simulink model "HvacMdlAirMFlowFrnt"  (R2022b)
settings: Solver=FixedStepDiscrete  StartTime=0.0  StopTime=10.0  FixedStep=0.2  RelTol=1e-3
settings Model: Creator=blue.ge  LastModifiedBy=blue.ge
settings DataIOCC: SaveFormat=StructureWithTime
settings OptimizationCC: DefaultParameterBehavior=Inlined  EnhancedBackFolding=on  PassReuseOutputArgsAs=Individual arguments  ZeroExternalMemoryAtStartup=off  ZeroInternalMemoryAtStartup=off  OptimizeBlockOrder=speed  DifferentSizesBufferReuse=on  OptimizationCustomize=off  EfficientTunableParamExpr=on
settings DebuggingCC: ParameterTunabilityLossMsg=error
settings ModelReferenceCC: ModelReferenceNumInstancesAllowed=Single
settings SFSimCC: MATLABDynamicMemAlloc=off
settings RTWCC: Description=AUTOSAR  SystemTargetFile=autosar.tlc  GenCodeOnly=on  TemplateMakefile=ert_default_tmf
settings CodeAppCC: ForceParamTrailComments=on  IgnoreCustomStorageClasses=off  OperatorAnnotations=on  SimulinkDataObjDesc=on  SFDataObjDesc=on  InsertBlockDesc=on
settings STFCustomTargetCC: TargetLangStandard=C99 (ISO)  MultiwordLength=256  ParMdlRefBuildCompliant=on  CompOptLevelCompliant=on  IncludeMdlTerminateFcn=off  CombineOutputUpdateFcns=on  SuppressErrorStatus=on  ERTCustomFileBanners=on  SupportNonFinite=off  SupportComplex=off  SupportContinuousTime=off  SupportNonInlinedSFcns=off  RemoveResetFunc=on  MATLABClassNameForMDSCustomization=Simulink.SoftwareTarget.ERTCustomization  AutosarCompliant=on  UseToolchainInfoCompliant=on  LookupTableObjectStructAxisOrder=2,1,3,4,...  LUTObjectStructOrderEvenSpacing=Size,Table,Breakpoints  SystemTargetFile=autosar.tlc
settings ERTTargetCC: TargetLangStandard=C99 (ISO)  ConcurrentExecutionCompliant=off  IncludeMdlTerminateFcn=off  SuppressErrorStatus=on  SupportNonFinite=off  SupportComplex=off  ModelStepFunctionPrototypeControlCompliant=off  CPPClassGenCompliant=off  AutosarCompliant=on  LookupTableObjectStructAxisOrder=2,1,3,4,...  LUTObjectStructOrderEvenSpacing=Size,Table,Breakpoints  InlinedParameterPlacement=NonHierarchical  ERTCustomFileTemplate=example_file_process.tlc

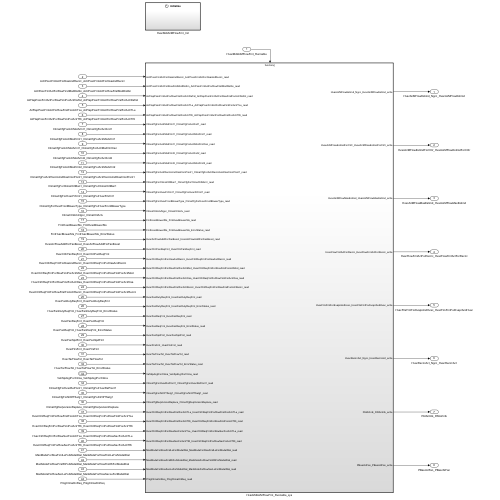
[thumbnail: Simulink's stored preview of the top-level diagram]
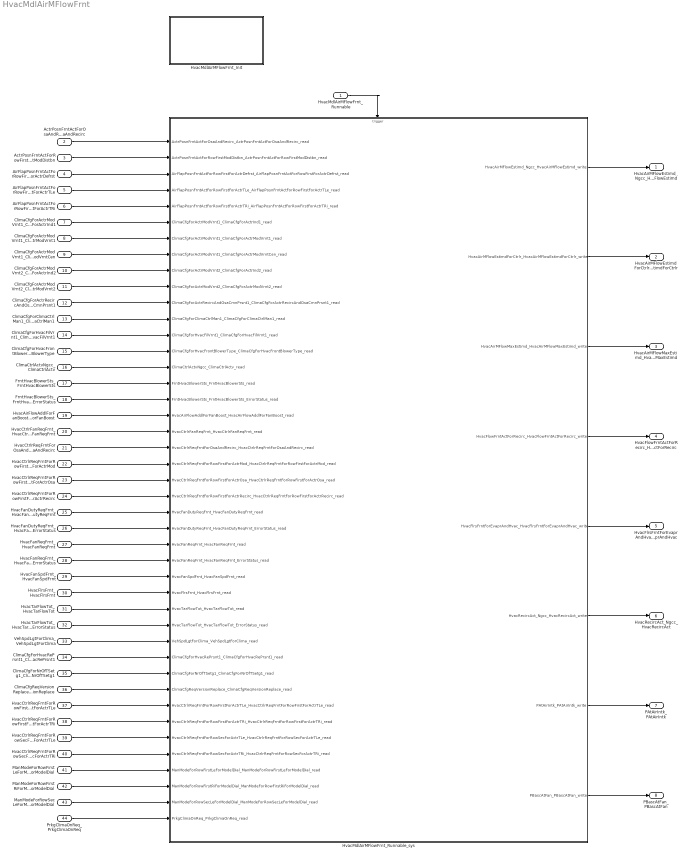
== system HvacMdlAirMFlowFrnt ==
  HvacMdlAirMFlowFrnt_Runnable : Inport  SampleTime=0.2  OutputFunctionCall=on
  ActrPosnFrntActForOsaAndRecirc_ActrPosnFrntActForOsaAndRecirc : Inport  Port=2  OutMin=0  OutMax=100  OutDataTypeStr=Perc9  PortDimensions=1
  ActrPosnFrntActForRowFirstModDistbn_ActrPosnFrntActForRowFirstModDistbn : Inport  Port=3  OutMin=0  OutMax=100  OutDataTypeStr=Perc9  PortDimensions=1
  AirFlapPosnFrntActForRowFirstForActrDefrst_AirFlapPosnFrntActForRowFirstForActrDefrst : Inport  Port=4  OutMin=0  OutMax=100  OutDataTypeStr=Perc9  PortDimensions=1
  AirFlapPosnFrntActForRowFirstForActrTLe_AirFlapPosnFrntActForRowFirstForActrTLe : Inport  Port=5  OutMin=0  OutMax=100  OutDataTypeStr=Perc9  PortDimensions=1
  AirFlapPosnFrntActForRowFirstForActrTRi_AirFlapPosnFrntActForRowFirstForActrTRi : Inport  Port=6  OutMin=0  OutMax=100  OutDataTypeStr=Perc9  PortDimensions=1
  ClimaCfgForActrModVrnt1_ClimaCfgForActrInd1 : Inport  Port=7  OutDataTypeStr=Enum: ClimaCfgForActrInd1  PortDimensions=1
  ClimaCfgForActrModVrnt1_ClimaCfgForActrModVrnt1 : Inport  Port=8  OutDataTypeStr=Enum: ClimaCfgForActrModVrnt1  PortDimensions=1
  ClimaCfgForActrModVrnt1_ClimaCfgForActrModVrntCen : Inport  Port=9  OutDataTypeStr=Enum: ClimaCfgForActrModVrntCen  PortDimensions=1
  ClimaCfgForActrModVrnt2_ClimaCfgForActrInd2 : Inport  Port=10  OutDataTypeStr=Enum: ClimaCfgForActrInd2  PortDimensions=1
  ClimaCfgForActrModVrnt2_ClimaCfgForActrModVrnt2 : Inport  Port=11  OutDataTypeStr=Enum: ClimaCfgForActrModVrnt2  PortDimensions=1
  ClimaCfgForActrRecircAndOsaCmnPrsnt1_ClimaCfgForActrRecircAndOsaCmnPrsnt1 : Inport  Port=12  OutDataTypeStr=Enum: ClimaCfgForActrRecircAndOsaCmnPrsnt1  PortDimensions=1
  ClimaCfgForClimaCtrlMan1_ClimaCfgForClimaCtrlMan1 : Inport  Port=13  OutDataTypeStr=Boolean  PortDimensions=1
  ClimaCfgForHvacFilVrnt1_ClimaCfgForHvacFilVrnt1 : Inport  Port=14  OutDataTypeStr=Enum: CarCfgForHvacFilTypGly  PortDimensions=1
  ClimaCfgForHvacFrontBlowerType_ClimaCfgForHvacFrontBlowerType : Inport  Port=15  OutDataTypeStr=Enum: ClimaCfgForHvacFrontBlowerType  PortDimensions=1
  ClimaCtrlActvNgcc_ClimaCtrlActv : Inport  Port=16  OutDataTypeStr=Boolean  PortDimensions=1
  FrntHvacBlowerSts_FrntHvacBlowerSts : Inport  Port=17  OutDataTypeStr=Enum: HvacFanSts  PortDimensions=1
  FrntHvacBlowerSts_FrntHvacBlowerSts_ErrorStatus : Inport  Port=18  OutDataTypeStr=uint8  PortDimensions=1
  HvacAirFlowAddlForFanBoost_HvacAirFlowAddlForFanBoost : Inport  Port=19  OutDataTypeStr=AirFlow30  PortDimensions=1
  HvacCtrlrFanReqFrnt_HvacCtrlrFanReqFrnt : Inport  Port=20  OutDataTypeStr=FanSpd1  PortDimensions=1
  HvacCtrlrReqFrntForOsaAndRecirc_HvacCtrlrReqFrntForOsaAndRecirc : Inport  Port=21  OutDataTypeStr=Perc30  PortDimensions=1
  HvacCtrlrReqFrntForRowFirstForActrMod_HvacCtrlrReqFrntForRowFirstForActrMod : Inport  Port=22  OutDataTypeStr=Perc30  PortDimensions=1
  HvacCtrlrReqFrntForRowFirstForActrOsa_HvacCtrlrReqFrntForRowFirstForActrOsa : Inport  Port=23  OutDataTypeStr=Perc30  PortDimensions=1
  HvacCtrlrReqFrntForRowFirstForActrRecirc_HvacCtrlrReqFrntForRowFirstForActrRecirc : Inport  Port=24  OutDataTypeStr=Perc30  PortDimensions=1
  HvacFanDutyReqFrnt_HvacFanDutyReqFrnt : Inport  Port=25  OutMin=0  OutMax=100  OutDataTypeStr=Perc9  PortDimensions=1
  HvacFanDutyReqFrnt_HvacFanDutyReqFrnt_ErrorStatus : Inport  Port=26  OutDataTypeStr=uint8  PortDimensions=1
  HvacFanReqFrnt_HvacFanReqFrnt : Inport  Port=27  OutDataTypeStr=HvacFanSpd  PortDimensions=1
  HvacFanReqFrnt_HvacFanReqFrnt_ErrorStatus : Inport  Port=28  OutDataTypeStr=uint8  PortDimensions=1
  HvacFanSpdFrnt_HvacFanSpdFrnt : Inport  Port=29  OutDataTypeStr=HvacFanSpd  PortDimensions=1
  HvacFlrsFrnt_HvacFlrsFrnt : Inport  Port=30  OutDataTypeStr=Flrs30  PortDimensions=1
  HvacTarFlowTot_HvacTarFlowTot : Inport  Port=31  OutDataTypeStr=AirFlow30  PortDimensions=1
  HvacTarFlowTot_HvacTarFlowTot_ErrorStatus : Inport  Port=32  OutDataTypeStr=uint8  PortDimensions=1
  VehSpdLgtForClima_VehSpdLgtForClima : Inport  Port=33  OutDataTypeStr=Bus: VehSpdLgtForClima  PortDimensions=1  BusOutputAsStruct=on
  ClimaCfgForHvacRePrsnt1_ClimaCfgForHvacRePrsnt1 : Inport  Port=34  OutDataTypeStr=Enum: CarCfgForHvacRe  PortDimensions=1
  ClimaCfgForNrOfTSetg1_ClimaCfgForNrOfTSetg1 : Inport  Port=35  OutDataTypeStr=Enum: ClimaCfgForNrOfTSetg1  PortDimensions=1
  ClimaCfgReqVersionReplace_ClimaCfgReqVersionReplace : Inport  Port=36  OutDataTypeStr=UInt8  PortDimensions=1
  HvacCtrlrReqFrntForRowFirstForActrTLe_HvacCtrlrReqFrntForRowFirstForActrTLe : Inport  Port=37  OutDataTypeStr=Perc30  PortDimensions=1
  HvacCtrlrReqFrntForRowFirstForActrTRi_HvacCtrlrReqFrntForRowFirstForActrTRi : Inport  Port=38  OutDataTypeStr=Perc30  PortDimensions=1
  HvacCtrlrReqFrntForRowSecForActrTLe_HvacCtrlrReqFrntForRowSecForActrTLe : Inport  Port=39  OutDataTypeStr=Perc30  PortDimensions=1
  HvacCtrlrReqFrntForRowSecForActrTRi_HvacCtrlrReqFrntForRowSecForActrTRi : Inport  Port=40  OutDataTypeStr=Perc30  PortDimensions=1
  ManModeForRowFirstLeForModelDial_ManModeForRowFirstLeForModelDial : Inport  Port=41  OutDataTypeStr=Enum: HmiCmptmtAirDistbnFrnt  PortDimensions=1
  ManModeForRowFirstRiForModelDial_ManModeForRowFirstRiForModelDial : Inport  Port=42  OutDataTypeStr=Enum: HmiCmptmtAirDistbnFrnt  PortDimensions=1
  ManModeForRowSecLeForModelDial_ManModeForRowSecLeForModelDial : Inport  Port=43  OutDataTypeStr=Enum: HmiCmptmtAirDistbnFrnt  PortDimensions=1
  PrkgClimaOnReq_PrkgClimaOnReq : Inport  Port=44  OutDataTypeStr=Boolean  PortDimensions=1
  HvacMdlAirMFlowFrnt_Init : Subsystem
  HvacMdlAirMFlowFrnt_Runnable_sys : Subsystem
  HvacAirMFlowEstimd_Ngcc_HvacAirMFlowEstimd : Outport  OutMin=0  OutMax=1000  OutDataTypeStr=MFlow1  PortDimensions=1
  HvacAirMFlowEstimdForCtrlr_HvacAirMFlowEstimdForCtrlr : Outport  Port=2  OutDataTypeStr=AirFlow30  PortDimensions=1
  HvacAirMFlowMaxEstimd_HvacAirMFlowMaxEstimd : Outport  Port=3  OutDataTypeStr=AirFlow30  PortDimensions=1
  HvacFlowFrntActForRecirc_HvacFlowFrntActForRecirc : Outport  Port=4  OutDataTypeStr=AirFlow30  PortDimensions=1
  HvacFlrsFrntForEvaprAndHvac_HvacFlrsFrntForEvaprAndHvac : Outport  Port=5  OutDataTypeStr=Flrs30  PortDimensions=1
  HvacRecircAct_Ngcc_HvacRecircAct : Outport  Port=6  OutDataTypeStr=Perc4  PortDimensions=1
  PAtAirIntk_PAtAirIntk : Outport  Port=7  OutDataTypeStr=AirP31  PortDimensions=1
  PBascAtFan_PBascAtFan : Outport  Port=8  OutDataTypeStr=AirP31  PortDimensions=1
  wires:
  ActrPosnFrntActForOsaAndRecirc_ActrPosnFrntActForOsaAndRecirc/1 -> HvacMdlAirMFlowFrnt_Runnable_sys/1(ActrPosnFrntActForOsaAndRecirc_ActrPosnFrntActForOsaAndRecirc_read)
  ActrPosnFrntActForRowFirstModDistbn_ActrPosnFrntActForRowFirstModDistbn/1 -> HvacMdlAirMFlowFrnt_Runnable_sys/2(ActrPosnFrntActForRowFirstModDistbn_ActrPosnFrntActForRowFirstModDistbn_read)
  AirFlapPosnFrntActForRowFirstForActrDefrst_AirFlapPosnFrntActForRowFirstForActrDefrst/1 -> HvacMdlAirMFlowFrnt_Runnable_sys/3(AirFlapPosnFrntActForRowFirstForActrDefrst_AirFlapPosnFrntActForRowFirstForActrDefrst_read)
  AirFlapPosnFrntActForRowFirstForActrTLe_AirFlapPosnFrntActForRowFirstForActrTLe/1 -> HvacMdlAirMFlowFrnt_Runnable_sys/4(AirFlapPosnFrntActForRowFirstForActrTLe_AirFlapPosnFrntActForRowFirstForActrTLe_read)
  AirFlapPosnFrntActForRowFirstForActrTRi_AirFlapPosnFrntActForRowFirstForActrTRi/1 -> HvacMdlAirMFlowFrnt_Runnable_sys/5(AirFlapPosnFrntActForRowFirstForActrTRi_AirFlapPosnFrntActForRowFirstForActrTRi_read)
  ClimaCfgForActrModVrnt1_ClimaCfgForActrInd1/1 -> HvacMdlAirMFlowFrnt_Runnable_sys/6(ClimaCfgForActrModVrnt1_ClimaCfgForActrInd1_read)
  ClimaCfgForActrModVrnt1_ClimaCfgForActrModVrnt1/1 -> HvacMdlAirMFlowFrnt_Runnable_sys/7(ClimaCfgForActrModVrnt1_ClimaCfgForActrModVrnt1_read)
  ClimaCfgForActrModVrnt1_ClimaCfgForActrModVrntCen/1 -> HvacMdlAirMFlowFrnt_Runnable_sys/8(ClimaCfgForActrModVrnt1_ClimaCfgForActrModVrntCen_read)
  ClimaCfgForActrModVrnt2_ClimaCfgForActrInd2/1 -> HvacMdlAirMFlowFrnt_Runnable_sys/9(ClimaCfgForActrModVrnt2_ClimaCfgForActrInd2_read)
  ClimaCfgForActrModVrnt2_ClimaCfgForActrModVrnt2/1 -> HvacMdlAirMFlowFrnt_Runnable_sys/10(ClimaCfgForActrModVrnt2_ClimaCfgForActrModVrnt2_read)
  ClimaCfgForActrRecircAndOsaCmnPrsnt1_ClimaCfgForActrRecircAndOsaCmnPrsnt1/1 -> HvacMdlAirMFlowFrnt_Runnable_sys/11(ClimaCfgForActrRecircAndOsaCmnPrsnt1_ClimaCfgForActrRecircAndOsaCmnPrsnt1_read)
  ClimaCfgForClimaCtrlMan1_ClimaCfgForClimaCtrlMan1/1 -> HvacMdlAirMFlowFrnt_Runnable_sys/12(ClimaCfgForClimaCtrlMan1_ClimaCfgForClimaCtrlMan1_read)
  ClimaCfgForHvacFilVrnt1_ClimaCfgForHvacFilVrnt1/1 -> HvacMdlAirMFlowFrnt_Runnable_sys/13(ClimaCfgForHvacFilVrnt1_ClimaCfgForHvacFilVrnt1_read)
  ClimaCfgForHvacFrontBlowerType_ClimaCfgForHvacFrontBlowerType/1 -> HvacMdlAirMFlowFrnt_Runnable_sys/14(ClimaCfgForHvacFrontBlowerType_ClimaCfgForHvacFrontBlowerType_read)
  ClimaCtrlActvNgcc_ClimaCtrlActv/1 -> HvacMdlAirMFlowFrnt_Runnable_sys/15(ClimaCtrlActvNgcc_ClimaCtrlActv_read)
  FrntHvacBlowerSts_FrntHvacBlowerSts/1 -> HvacMdlAirMFlowFrnt_Runnable_sys/16(FrntHvacBlowerSts_FrntHvacBlowerSts_read)
  FrntHvacBlowerSts_FrntHvacBlowerSts_ErrorStatus/1 -> HvacMdlAirMFlowFrnt_Runnable_sys/17(FrntHvacBlowerSts_FrntHvacBlowerSts_ErrorStatus_read)
  HvacAirFlowAddlForFanBoost_HvacAirFlowAddlForFanBoost/1 -> HvacMdlAirMFlowFrnt_Runnable_sys/18(HvacAirFlowAddlForFanBoost_HvacAirFlowAddlForFanBoost_read)
  HvacCtrlrFanReqFrnt_HvacCtrlrFanReqFrnt/1 -> HvacMdlAirMFlowFrnt_Runnable_sys/19(HvacCtrlrFanReqFrnt_HvacCtrlrFanReqFrnt_read)
  HvacCtrlrReqFrntForOsaAndRecirc_HvacCtrlrReqFrntForOsaAndRecirc/1 -> HvacMdlAirMFlowFrnt_Runnable_sys/20(HvacCtrlrReqFrntForOsaAndRecirc_HvacCtrlrReqFrntForOsaAndRecirc_read)
  HvacCtrlrReqFrntForRowFirstForActrMod_HvacCtrlrReqFrntForRowFirstForActrMod/1 -> HvacMdlAirMFlowFrnt_Runnable_sys/21(HvacCtrlrReqFrntForRowFirstForActrMod_HvacCtrlrReqFrntForRowFirstForActrMod_read)
  HvacCtrlrReqFrntForRowFirstForActrOsa_HvacCtrlrReqFrntForRowFirstForActrOsa/1 -> HvacMdlAirMFlowFrnt_Runnable_sys/22(HvacCtrlrReqFrntForRowFirstForActrOsa_HvacCtrlrReqFrntForRowFirstForActrOsa_read)
  HvacCtrlrReqFrntForRowFirstForActrRecirc_HvacCtrlrReqFrntForRowFirstForActrRecirc/1 -> HvacMdlAirMFlowFrnt_Runnable_sys/23(HvacCtrlrReqFrntForRowFirstForActrRecirc_HvacCtrlrReqFrntForRowFirstForActrRecirc_read)
  HvacFanDutyReqFrnt_HvacFanDutyReqFrnt/1 -> HvacMdlAirMFlowFrnt_Runnable_sys/24(HvacFanDutyReqFrnt_HvacFanDutyReqFrnt_read)
  HvacFanDutyReqFrnt_HvacFanDutyReqFrnt_ErrorStatus/1 -> HvacMdlAirMFlowFrnt_Runnable_sys/25(HvacFanDutyReqFrnt_HvacFanDutyReqFrnt_ErrorStatus_read)
  HvacFanReqFrnt_HvacFanReqFrnt/1 -> HvacMdlAirMFlowFrnt_Runnable_sys/26(HvacFanReqFrnt_HvacFanReqFrnt_read)
  HvacFanReqFrnt_HvacFanReqFrnt_ErrorStatus/1 -> HvacMdlAirMFlowFrnt_Runnable_sys/27(HvacFanReqFrnt_HvacFanReqFrnt_ErrorStatus_read)
  HvacFanSpdFrnt_HvacFanSpdFrnt/1 -> HvacMdlAirMFlowFrnt_Runnable_sys/28(HvacFanSpdFrnt_HvacFanSpdFrnt_read)
  HvacFlrsFrnt_HvacFlrsFrnt/1 -> HvacMdlAirMFlowFrnt_Runnable_sys/29(HvacFlrsFrnt_HvacFlrsFrnt_read)
  HvacTarFlowTot_HvacTarFlowTot/1 -> HvacMdlAirMFlowFrnt_Runnable_sys/30(HvacTarFlowTot_HvacTarFlowTot_read)
  HvacTarFlowTot_HvacTarFlowTot_ErrorStatus/1 -> HvacMdlAirMFlowFrnt_Runnable_sys/31(HvacTarFlowTot_HvacTarFlowTot_ErrorStatus_read)
  VehSpdLgtForClima_VehSpdLgtForClima/1 -> HvacMdlAirMFlowFrnt_Runnable_sys/32(VehSpdLgtForClima_VehSpdLgtForClima_read)
  ClimaCfgForHvacRePrsnt1_ClimaCfgForHvacRePrsnt1/1 -> HvacMdlAirMFlowFrnt_Runnable_sys/33(ClimaCfgForHvacRePrsnt1_ClimaCfgForHvacRePrsnt1_read)
  ClimaCfgForNrOfTSetg1_ClimaCfgForNrOfTSetg1/1 -> HvacMdlAirMFlowFrnt_Runnable_sys/34(ClimaCfgForNrOfTSetg1_ClimaCfgForNrOfTSetg1_read)
  ClimaCfgReqVersionReplace_ClimaCfgReqVersionReplace/1 -> HvacMdlAirMFlowFrnt_Runnable_sys/35(ClimaCfgReqVersionReplace_ClimaCfgReqVersionReplace_read)
  HvacCtrlrReqFrntForRowFirstForActrTLe_HvacCtrlrReqFrntForRowFirstForActrTLe/1 -> HvacMdlAirMFlowFrnt_Runnable_sys/36(HvacCtrlrReqFrntForRowFirstForActrTLe_HvacCtrlrReqFrntForRowFirstForActrTLe_read)
  HvacCtrlrReqFrntForRowFirstForActrTRi_HvacCtrlrReqFrntForRowFirstForActrTRi/1 -> HvacMdlAirMFlowFrnt_Runnable_sys/37(HvacCtrlrReqFrntForRowFirstForActrTRi_HvacCtrlrReqFrntForRowFirstForActrTRi_read)
  HvacCtrlrReqFrntForRowSecForActrTLe_HvacCtrlrReqFrntForRowSecForActrTLe/1 -> HvacMdlAirMFlowFrnt_Runnable_sys/38(HvacCtrlrReqFrntForRowSecForActrTLe_HvacCtrlrReqFrntForRowSecForActrTLe_read)
  HvacCtrlrReqFrntForRowSecForActrTRi_HvacCtrlrReqFrntForRowSecForActrTRi/1 -> HvacMdlAirMFlowFrnt_Runnable_sys/39(HvacCtrlrReqFrntForRowSecForActrTRi_HvacCtrlrReqFrntForRowSecForActrTRi_read)
  ManModeForRowFirstLeForModelDial_ManModeForRowFirstLeForModelDial/1 -> HvacMdlAirMFlowFrnt_Runnable_sys/40(ManModeForRowFirstLeForModelDial_ManModeForRowFirstLeForModelDial_read)
  ManModeForRowFirstRiForModelDial_ManModeForRowFirstRiForModelDial/1 -> HvacMdlAirMFlowFrnt_Runnable_sys/41(ManModeForRowFirstRiForModelDial_ManModeForRowFirstRiForModelDial_read)
  ManModeForRowSecLeForModelDial_ManModeForRowSecLeForModelDial/1 -> HvacMdlAirMFlowFrnt_Runnable_sys/42(ManModeForRowSecLeForModelDial_ManModeForRowSecLeForModelDial_read)
  PrkgClimaOnReq_PrkgClimaOnReq/1 -> HvacMdlAirMFlowFrnt_Runnable_sys/43(PrkgClimaOnReq_PrkgClimaOnReq_read)
  HvacMdlAirMFlowFrnt_Runnable_sys/1(HvacAirMFlowEstimd_Ngcc_HvacAirMFlowEstimd_write) -> HvacAirMFlowEstimd_Ngcc_HvacAirMFlowEstimd/1
  HvacMdlAirMFlowFrnt_Runnable_sys/2(HvacAirMFlowEstimdForCtrlr_HvacAirMFlowEstimdForCtrlr_write) -> HvacAirMFlowEstimdForCtrlr_HvacAirMFlowEstimdForCtrlr/1
  HvacMdlAirMFlowFrnt_Runnable_sys/3(HvacAirMFlowMaxEstimd_HvacAirMFlowMaxEstimd_write) -> HvacAirMFlowMaxEstimd_HvacAirMFlowMaxEstimd/1
  HvacMdlAirMFlowFrnt_Runnable_sys/4(HvacFlowFrntActForRecirc_HvacFlowFrntActForRecirc_write) -> HvacFlowFrntActForRecirc_HvacFlowFrntActForRecirc/1
  HvacMdlAirMFlowFrnt_Runnable_sys/5(HvacFlrsFrntForEvaprAndHvac_HvacFlrsFrntForEvaprAndHvac_write) -> HvacFlrsFrntForEvaprAndHvac_HvacFlrsFrntForEvaprAndHvac/1
  HvacMdlAirMFlowFrnt_Runnable_sys/6(HvacRecircAct_Ngcc_HvacRecircAct_write) -> HvacRecircAct_Ngcc_HvacRecircAct/1
  HvacMdlAirMFlowFrnt_Runnable_sys/7(PAtAirIntk_PAtAirIntk_write) -> PAtAirIntk_PAtAirIntk/1
  HvacMdlAirMFlowFrnt_Runnable_sys/8(PBascAtFan_PBascAtFan_write) -> PBascAtFan_PBascAtFan/1
  HvacMdlAirMFlowFrnt_Runnable/1 -> HvacMdlAirMFlowFrnt_Runnable_sys/Trigger

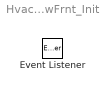
== system HvacMdlAirMFlowFrnt/HvacMdlAirMFlowFrnt_Init ==
  Event Listener : EventListener  EventName=reset

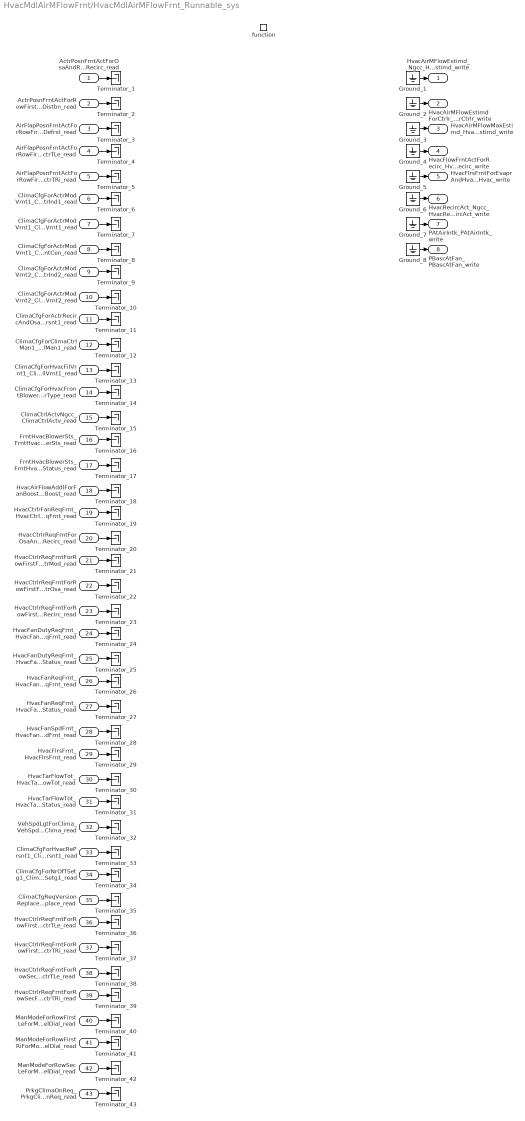
== system HvacMdlAirMFlowFrnt/HvacMdlAirMFlowFrnt_Runnable_sys ==
  ActrPosnFrntActForOsaAndRecirc_ActrPosnFrntActForOsaAndRecirc_read : Inport
  ActrPosnFrntActForRowFirstModDistbn_ActrPosnFrntActForRowFirstModDistbn_read : Inport  Port=2
  AirFlapPosnFrntActForRowFirstForActrDefrst_AirFlapPosnFrntActForRowFirstForActrDefrst_read : Inport  Port=3
  AirFlapPosnFrntActForRowFirstForActrTLe_AirFlapPosnFrntActForRowFirstForActrTLe_read : Inport  Port=4
  AirFlapPosnFrntActForRowFirstForActrTRi_AirFlapPosnFrntActForRowFirstForActrTRi_read : Inport  Port=5
  ClimaCfgForActrModVrnt1_ClimaCfgForActrInd1_read : Inport  Port=6
  ClimaCfgForActrModVrnt1_ClimaCfgForActrModVrnt1_read : Inport  Port=7
  ClimaCfgForActrModVrnt1_ClimaCfgForActrModVrntCen_read : Inport  Port=8
  ClimaCfgForActrModVrnt2_ClimaCfgForActrInd2_read : Inport  Port=9
  ClimaCfgForActrModVrnt2_ClimaCfgForActrModVrnt2_read : Inport  Port=10
  ClimaCfgForActrRecircAndOsaCmnPrsnt1_ClimaCfgForActrRecircAndOsaCmnPrsnt1_read : Inport  Port=11
  ClimaCfgForClimaCtrlMan1_ClimaCfgForClimaCtrlMan1_read : Inport  Port=12
  ClimaCfgForHvacFilVrnt1_ClimaCfgForHvacFilVrnt1_read : Inport  Port=13
  ClimaCfgForHvacFrontBlowerType_ClimaCfgForHvacFrontBlowerType_read : Inport  Port=14
  ClimaCtrlActvNgcc_ClimaCtrlActv_read : Inport  Port=15
  FrntHvacBlowerSts_FrntHvacBlowerSts_read : Inport  Port=16
  FrntHvacBlowerSts_FrntHvacBlowerSts_ErrorStatus_read : Inport  Port=17
  HvacAirFlowAddlForFanBoost_HvacAirFlowAddlForFanBoost_read : Inport  Port=18
  HvacCtrlrFanReqFrnt_HvacCtrlrFanReqFrnt_read : Inport  Port=19
  HvacCtrlrReqFrntForOsaAndRecirc_HvacCtrlrReqFrntForOsaAndRecirc_read : Inport  Port=20
  HvacCtrlrReqFrntForRowFirstForActrMod_HvacCtrlrReqFrntForRowFirstForActrMod_read : Inport  Port=21
  HvacCtrlrReqFrntForRowFirstForActrOsa_HvacCtrlrReqFrntForRowFirstForActrOsa_read : Inport  Port=22
  HvacCtrlrReqFrntForRowFirstForActrRecirc_HvacCtrlrReqFrntForRowFirstForActrRecirc_read : Inport  Port=23
  HvacFanDutyReqFrnt_HvacFanDutyReqFrnt_read : Inport  Port=24
  HvacFanDutyReqFrnt_HvacFanDutyReqFrnt_ErrorStatus_read : Inport  Port=25
  HvacFanReqFrnt_HvacFanReqFrnt_read : Inport  Port=26
  HvacFanReqFrnt_HvacFanReqFrnt_ErrorStatus_read : Inport  Port=27
  HvacFanSpdFrnt_HvacFanSpdFrnt_read : Inport  Port=28
  HvacFlrsFrnt_HvacFlrsFrnt_read : Inport  Port=29
  HvacTarFlowTot_HvacTarFlowTot_read : Inport  Port=30
  HvacTarFlowTot_HvacTarFlowTot_ErrorStatus_read : Inport  Port=31
  VehSpdLgtForClima_VehSpdLgtForClima_read : Inport  Port=32
  ClimaCfgForHvacRePrsnt1_ClimaCfgForHvacRePrsnt1_read : Inport  Port=33
  ClimaCfgForNrOfTSetg1_ClimaCfgForNrOfTSetg1_read : Inport  Port=34
  ClimaCfgReqVersionReplace_ClimaCfgReqVersionReplace_read : Inport  Port=35
  HvacCtrlrReqFrntForRowFirstForActrTLe_HvacCtrlrReqFrntForRowFirstForActrTLe_read : Inport  Port=36
  HvacCtrlrReqFrntForRowFirstForActrTRi_HvacCtrlrReqFrntForRowFirstForActrTRi_read : Inport  Port=37
  HvacCtrlrReqFrntForRowSecForActrTLe_HvacCtrlrReqFrntForRowSecForActrTLe_read : Inport  Port=38
  HvacCtrlrReqFrntForRowSecForActrTRi_HvacCtrlrReqFrntForRowSecForActrTRi_read : Inport  Port=39
  ManModeForRowFirstLeForModelDial_ManModeForRowFirstLeForModelDial_read : Inport  Port=40
  ManModeForRowFirstRiForModelDial_ManModeForRowFirstRiForModelDial_read : Inport  Port=41
  ManModeForRowSecLeForModelDial_ManModeForRowSecLeForModelDial_read : Inport  Port=42
  PrkgClimaOnReq_PrkgClimaOnReq_read : Inport  Port=43
  function : TriggerPort  TriggerType=function-call  VariantControl=(inherit)  SampleTimeType=periodic  SampleTime=-1
  Ground_{1..8} (8 alike) : Ground
  Terminator_{1..43} (43 alike) : Terminator
  HvacAirMFlowEstimd_Ngcc_HvacAirMFlowEstimd_write : Outport
      settings: EnsureOutportIsVirtual=on
  HvacAirMFlowEstimdForCtrlr_HvacAirMFlowEstimdForCtrlr_write : Outport  Port=2
      settings: EnsureOutportIsVirtual=on
  HvacAirMFlowMaxEstimd_HvacAirMFlowMaxEstimd_write : Outport  Port=3
      settings: EnsureOutportIsVirtual=on
  HvacFlowFrntActForRecirc_HvacFlowFrntActForRecirc_write : Outport  Port=4
      settings: EnsureOutportIsVirtual=on
  HvacFlrsFrntForEvaprAndHvac_HvacFlrsFrntForEvaprAndHvac_write : Outport  Port=5
      settings: EnsureOutportIsVirtual=on
  HvacRecircAct_Ngcc_HvacRecircAct_write : Outport  Port=6
      settings: EnsureOutportIsVirtual=on
  PAtAirIntk_PAtAirIntk_write : Outport  Port=7
      settings: EnsureOutportIsVirtual=on
  PBascAtFan_PBascAtFan_write : Outport  Port=8
      settings: EnsureOutportIsVirtual=on
  wires:
  ActrPosnFrntActForOsaAndRecirc_ActrPosnFrntActForOsaAndRecirc_read/1 -> Terminator_1/1
  ActrPosnFrntActForRowFirstModDistbn_ActrPosnFrntActForRowFirstModDistbn_read/1 -> Terminator_2/1
  AirFlapPosnFrntActForRowFirstForActrDefrst_AirFlapPosnFrntActForRowFirstForActrDefrst_read/1 -> Terminator_3/1
  AirFlapPosnFrntActForRowFirstForActrTLe_AirFlapPosnFrntActForRowFirstForActrTLe_read/1 -> Terminator_4/1
  AirFlapPosnFrntActForRowFirstForActrTRi_AirFlapPosnFrntActForRowFirstForActrTRi_read/1 -> Terminator_5/1
  ClimaCfgForActrModVrnt1_ClimaCfgForActrInd1_read/1 -> Terminator_6/1
  ClimaCfgForActrModVrnt1_ClimaCfgForActrModVrnt1_read/1 -> Terminator_7/1
  ClimaCfgForActrModVrnt1_ClimaCfgForActrModVrntCen_read/1 -> Terminator_8/1
  ClimaCfgForActrModVrnt2_ClimaCfgForActrInd2_read/1 -> Terminator_9/1
  ClimaCfgForActrModVrnt2_ClimaCfgForActrModVrnt2_read/1 -> Terminator_10/1
  ClimaCfgForActrRecircAndOsaCmnPrsnt1_ClimaCfgForActrRecircAndOsaCmnPrsnt1_read/1 -> Terminator_11/1
  ClimaCfgForClimaCtrlMan1_ClimaCfgForClimaCtrlMan1_read/1 -> Terminator_12/1
  ClimaCfgForHvacFilVrnt1_ClimaCfgForHvacFilVrnt1_read/1 -> Terminator_13/1
  ClimaCfgForHvacFrontBlowerType_ClimaCfgForHvacFrontBlowerType_read/1 -> Terminator_14/1
  ClimaCtrlActvNgcc_ClimaCtrlActv_read/1 -> Terminator_15/1
  FrntHvacBlowerSts_FrntHvacBlowerSts_read/1 -> Terminator_16/1
  FrntHvacBlowerSts_FrntHvacBlowerSts_ErrorStatus_read/1 -> Terminator_17/1
  HvacAirFlowAddlForFanBoost_HvacAirFlowAddlForFanBoost_read/1 -> Terminator_18/1
  HvacCtrlrFanReqFrnt_HvacCtrlrFanReqFrnt_read/1 -> Terminator_19/1
  HvacCtrlrReqFrntForOsaAndRecirc_HvacCtrlrReqFrntForOsaAndRecirc_read/1 -> Terminator_20/1
  HvacCtrlrReqFrntForRowFirstForActrMod_HvacCtrlrReqFrntForRowFirstForActrMod_read/1 -> Terminator_21/1
  HvacCtrlrReqFrntForRowFirstForActrOsa_HvacCtrlrReqFrntForRowFirstForActrOsa_read/1 -> Terminator_22/1
  HvacCtrlrReqFrntForRowFirstForActrRecirc_HvacCtrlrReqFrntForRowFirstForActrRecirc_read/1 -> Terminator_23/1
  HvacFanDutyReqFrnt_HvacFanDutyReqFrnt_read/1 -> Terminator_24/1
  HvacFanDutyReqFrnt_HvacFanDutyReqFrnt_ErrorStatus_read/1 -> Terminator_25/1
  HvacFanReqFrnt_HvacFanReqFrnt_read/1 -> Terminator_26/1
  HvacFanReqFrnt_HvacFanReqFrnt_ErrorStatus_read/1 -> Terminator_27/1
  HvacFanSpdFrnt_HvacFanSpdFrnt_read/1 -> Terminator_28/1
  HvacFlrsFrnt_HvacFlrsFrnt_read/1 -> Terminator_29/1
  HvacTarFlowTot_HvacTarFlowTot_read/1 -> Terminator_30/1
  HvacTarFlowTot_HvacTarFlowTot_ErrorStatus_read/1 -> Terminator_31/1
  VehSpdLgtForClima_VehSpdLgtForClima_read/1 -> Terminator_32/1
  ClimaCfgForHvacRePrsnt1_ClimaCfgForHvacRePrsnt1_read/1 -> Terminator_33/1
  ClimaCfgForNrOfTSetg1_ClimaCfgForNrOfTSetg1_read/1 -> Terminator_34/1
  ClimaCfgReqVersionReplace_ClimaCfgReqVersionReplace_read/1 -> Terminator_35/1
  HvacCtrlrReqFrntForRowFirstForActrTLe_HvacCtrlrReqFrntForRowFirstForActrTLe_read/1 -> Terminator_36/1
  HvacCtrlrReqFrntForRowFirstForActrTRi_HvacCtrlrReqFrntForRowFirstForActrTRi_read/1 -> Terminator_37/1
  HvacCtrlrReqFrntForRowSecForActrTLe_HvacCtrlrReqFrntForRowSecForActrTLe_read/1 -> Terminator_38/1
  HvacCtrlrReqFrntForRowSecForActrTRi_HvacCtrlrReqFrntForRowSecForActrTRi_read/1 -> Terminator_39/1
  ManModeForRowFirstLeForModelDial_ManModeForRowFirstLeForModelDial_read/1 -> Terminator_40/1
  ManModeForRowFirstRiForModelDial_ManModeForRowFirstRiForModelDial_read/1 -> Terminator_41/1
  ManModeForRowSecLeForModelDial_ManModeForRowSecLeForModelDial_read/1 -> Terminator_42/1
  PrkgClimaOnReq_PrkgClimaOnReq_read/1 -> Terminator_43/1
  Ground_1/1 -> HvacAirMFlowEstimd_Ngcc_HvacAirMFlowEstimd_write/1
  Ground_2/1 -> HvacAirMFlowEstimdForCtrlr_HvacAirMFlowEstimdForCtrlr_write/1
  Ground_3/1 -> HvacAirMFlowMaxEstimd_HvacAirMFlowMaxEstimd_write/1
  Ground_4/1 -> HvacFlowFrntActForRecirc_HvacFlowFrntActForRecirc_write/1
  Ground_5/1 -> HvacFlrsFrntForEvaprAndHvac_HvacFlrsFrntForEvaprAndHvac_write/1
  Ground_6/1 -> HvacRecircAct_Ngcc_HvacRecircAct_write/1
  Ground_7/1 -> PAtAirIntk_PAtAirIntk_write/1
  Ground_8/1 -> PBascAtFan_PBascAtFan_write/1
